simulink model "cddDcmApp"  (R2022b)
settings: Solver=FixedStepDiscrete  StartTime=0.0  StopTime=10.0  FixedStep=0.02  RelTol=1e-3
undefined symbols (not defined in the file or in its folder): CCPFaultType, DcChlType, HFAChlType, IcuChannelType, MtrRoutStaEnum, P_SignalPartType, P_Std_ReturnType, PowerChlType, SrsAdChlType, VGAChlType
(part 4 of 13)

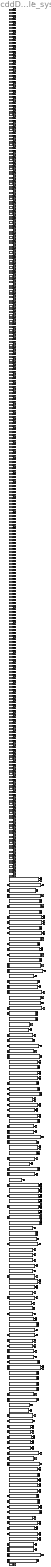
== system cddDcmApp/cddDcmApp_20ms_Runnable_sys ==
  AACAirClngErrStsNgcc_AACAirClngErrStsNgcc_read : Inport
  AACAirClngStsNgcc_AACAirClngStsNgcc_read : Inport  Port=2
  AfuFrameSts_AfuFrameSts_read : Inport  Port=3
  AFUGenErr_AFUGenErr_read : Inport  Port=4
  AirClngErrSts_AirClngErrSts_read : Inport  Port=5
  AirClngSts_AirClngSts_read : Inport  Port=6
  AirFragFanErr_AirFragFanErr_read : Inport  Port=7
  AirFragMot1Err_AirFragMot1Err_read : Inport  Port=8
  AirFragMot2Err_AirFragMot2Err_read : Inport  Port=9
  AirFragMot3Err_AirFragMot3Err_read : Inport  Port=10
  AirFragMot4Err_AirFragMot4Err_read : Inport  Port=11
  AirFragMot5Err_AirFragMot5Err_read : Inport  Port=12
  AirFragOvrVoltErr_AirFragOvrVoltErr_read : Inport  Port=13
  AirFragTempErr_AirFragTempErr_read : Inport  Port=14
  AirFragUndVoltErr_AirFragUndVoltErr_read : Inport  Port=15
  AirTFromHeatgEstimdFirstLeForCCM_AirTFromHeatgEstimdFirstLeForCCM_read : Inport  Port=16
  AirTFromHeatgEstimdFirstRiForCCM_AirTFromHeatgEstimdFirstRiForCCM_read : Inport  Port=17
  AirTFromHeatgEstimdForCCMForRead_AirTFromHeatgEstimdForCCMForRead_read : Inport  Port=18
  AirTFromHeatgEstimdReForCCM_AirTFromHeatgEstimdReForCCM_read : Inport  Port=19
  AirTFromHeatgEstimdSecLeForCCM_AirTFromHeatgEstimdSecLeForCCM_read : Inport  Port=20
  AiuFrameSts_AiuFrameSts_read : Inport  Port=21
  AmbTFildForClimaNgcc_AmbTFildForClima_read : Inport  Port=22
  AmbTRaw_AmbTRaw_read : Inport  Port=23
  AQSAirQuality_AQSAirQuality_read : Inport  Port=24
  AQSCOxstatus_AQSCOxstatus_read : Inport  Port=25
  AqsFrameSts_AqsFrameSts_read : Inport  Port=26
  AQSNH3status_AQSNH3status_read : Inport  Port=27
  AQSNoxStatus_AQSNoxStatus_read : Inport  Port=28
  BkpOfDstTrvld_BkpOfDstTrvld_read : Inport  Port=29
  BkpOfDstTrvld_BkpOfDstTrvld_IsUpdated_read : Inport  Port=30
  CarCfgForClimaCtrl_CarCfgForAirClng_read : Inport  Port=31
  CarCfgForClimaCtrl_CarCfgForAirClnrAdv_read : Inport  Port=32
  CarCfgForClimaCtrl_CarCfgForAirFrag_read : Inport  Port=33
  CarCfgForClimaCtrl_CarCfgForAirMtrlSnsr_read : Inport  Port=34
  CarCfgForClimaCtrl_CarCfgForAirQly_read : Inport  Port=35
  CarCfgForClimaCtrl_CarCfgForAmntOfSeat_read : Inport  Port=36
  CarCfgForClimaCtrl_CarCfgForAutDemst_read : Inport  Port=37
  CarCfgForClimaCtrl_CarCfgForAuxWtrPmp_read : Inport  Port=38
  CarCfgForClimaCtrl_CarCfgForBRAND_read : Inport  Port=39
  CarCfgForClimaCtrl_CarCfgForBypVlvInHvac_read : Inport  Port=40
  CarCfgForClimaCtrl_CarCfgForCabinOxgenSupply_read : Inport  Port=41
  CarCfgForClimaCtrl_CarCfgForClsVlvInHvac_read : Inport  Port=42
  CarCfgForClimaCtrl_CarCfgForCmprAcTyp_read : Inport  Port=43
  CarCfgForClimaCtrl_CarCfgForCmptmtClng_read : Inport  Port=44
  CarCfgForClimaCtrl_CarCfgForElectricLiftingModule_read : Inport  Port=45
  CarCfgForClimaCtrl_CarCfgForElecVentAdjmt_read : Inport  Port=46
  CarCfgForClimaCtrl_CarCfgForExtVentnClimaCtrl_read : Inport  Port=47
  CarCfgForClimaCtrl_CarCfgForFlcTyp_read : Inport  Port=48
  CarCfgForClimaCtrl_CarCfgForFloatingDriving_read : Inport  Port=49
  CarCfgForClimaCtrl_CarCfgForFoldCSD_read : Inport  Port=50
  CarCfgForClimaCtrl_CarCfgForFuTyp_read : Inport  Port=51
  CarCfgForClimaCtrl_CarCfgForGlassTyp_read : Inport  Port=52
  CarCfgForClimaCtrl_CarCfgForHeadRadar_read : Inport  Port=53
  CarCfgForClimaCtrl_CarCfgForHeatPmp_read : Inport  Port=54
  CarCfgForClimaCtrl_CarCfgForHeatrDurgDrvg_read : Inport  Port=55
  CarCfgForClimaCtrl_CarCfgForHeatrTyp_read : Inport  Port=56
  CarCfgForClimaCtrl_CarCfgForHvacFilTyp_read : Inport  Port=57
  CarCfgForClimaCtrl_CarCfgForHvacFlrSnsrForT_read : Inport  Port=58
  CarCfgForClimaCtrl_CarCfgForHvacHeatrSnsrT_read : Inport  Port=59
  CarCfgForClimaCtrl_CarCfgForHvacRe_read : Inport  Port=60
  CarCfgForClimaCtrl_CarCfgForHvacTyp_read : Inport  Port=61
  CarCfgForClimaCtrl_CarCfgForInteriorViewCamera_read : Inport  Port=62
  CarCfgForClimaCtrl_CarCfgForLifeDetection_read : Inport  Port=63
  CarCfgForClimaCtrl_CarCfgForLoVoltElecCfg_read : Inport  Port=64
  CarCfgForClimaCtrl_CarCfgForNavSysandIntnmap_read : Inport  Port=65
  CarCfgForClimaCtrl_CarCfgForNrOfDoor_read : Inport  Port=66
  CarCfgForClimaCtrl_CarCfgForPipeInFuTank_read : Inport  Port=67
  CarCfgForClimaCtrl_CarCfgForPowerSeats_read : Inport  Port=68
  CarCfgForClimaCtrl_CarCfgForPreClng_read : Inport  Port=69
  CarCfgForClimaCtrl_CarCfgForPrkgCDSNotAvl_read : Inport  Port=70
  CarCfgForClimaCtrl_CarCfgForPrkgClima_read : Inport  Port=71
  CarCfgForClimaCtrl_CarCfgForPrpsnTyp_read : Inport  Port=72
  CarCfgForClimaCtrl_CarCfgForPTCInFrontHVAC_read : Inport  Port=73
  CarCfgForClimaCtrl_CarCfgForRemEngStrtPreCdn_read : Inport  Port=74
  CarCfgForClimaCtrl_CarCfgForRoofHatch_read : Inport  Port=75
  CarCfgForClimaCtrl_CarCfgForSeatHeatgCal_read : Inport  Port=76
  CarCfgForClimaCtrl_CarCfgForSeatHeatgForRowFirst_read : Inport  Port=77
  CarCfgForClimaCtrl_CarCfgForSeatHeatgForRowSec_read : Inport  Port=78
  CarCfgForClimaCtrl_CarCfgForSeatVentnForRowFirst_read : Inport  Port=79
  CarCfgForClimaCtrl_CarCfgForSeatVentnForRowSec_read : Inport  Port=80
  CarCfgForClimaCtrl_CarCfgForSecRowVentnCenter_read : Inport  Port=81
  CarCfgForClimaCtrl_CarCfgForSecRowVentnSide_read : Inport  Port=82
  CarCfgForClimaCtrl_CarCfgForSmallFlowCyc_read : Inport  Port=83
  CarCfgForClimaCtrl_CarCfgForSmkSnsr_read : Inport  Port=84
  CarCfgForClimaCtrl_CarCfgForSnsrForCmptmtT_read : Inport  Port=85
  CarCfgForClimaCtrl_CarCfgForSnsrHumPrsnt_read : Inport  Port=86
  CarCfgForClimaCtrl_CarCfgForStartDevice_read : Inport  Port=87
  CarCfgForClimaCtrl_CarCfgForSteerWhlHeatg_read : Inport  Port=88
  CarCfgForClimaCtrl_CarCfgForSteerWhlPosn_read : Inport  Port=89
  CarCfgForClimaCtrl_CarCfgForSunSnsr_read : Inport  Port=90
  CarCfgForClimaCtrl_CarCfgForTelmNodTyp_read : Inport  Port=91
  CarCfgForClimaCtrl_CarCfgForTEMPEREDSTORAGEBOX_read : Inport  Port=92
  CarCfgForClimaCtrl_CarCfgForThermMngt_read : Inport  Port=93
  CarCfgForClimaCtrl_CarCfgForVehTyp_read : Inport  Port=94
  CarCfgForClimaCtrl_CarCfgForVentLiftMotorExiSts_read : Inport  Port=95
  CarCfgForClimaCtrl_CarCfgForVgaMotorExistSts_read : Inport  Port=96
  CarCfgForClimaCtrl_CarCfgForWadingAssistSystem_read : Inport  Port=97
  CarCfgForClimaCtrl_CarCfgForWindHeatd_read : Inport  Port=98
  CarCfgForClimaCtrl_CarCfgWindFollowHumanMov_read : Inport  Port=99
  CarTiGlb_CarTiGlb_read : Inport  Port=100
  CarTiGlb_CarTiGlb_IsUpdated_read : Inport  Port=101
  CcmIntCo2Lvl_CcmIntCo2Lvl_read : Inport  Port=102
  CCMLIN1frameSts_CCMLIN1frameSts_read : Inport  Port=103
  CCMLIN2frameSts_CCMLIN2frameSts_read : Inport  Port=104
  CCMLIN3frameSts_CCMLIN3frameSts_read : Inport  Port=105
  CdsFrameSts_CdsFrameSts_read : Inport  Port=106
  ClimaObsActv_ClimaObsActv_read : Inport  Port=107
  CmpmtInAirPmAQI_CmpmtInAirPmAQI_read : Inport  Port=108
  CmpmtInAirPmDnsty_CmpmtInAirPmDnsty_read : Inport  Port=109
  CmptmtAirTEstimdAtRowFirst_CmptmtAirTEstimdAtRowFirst_read : Inport  Port=110
  CmptmtAirTEstimdAtRowFirstIni_CmptmtAirTEstimdAtRowFirstIni_read : Inport  Port=111
  CmptmtAirTEstimdAtRowSec_CmptmtAirTEstimdAtRowSec_read : Inport  Port=112
  CmptmtAirTEstimdAtRowSecIni_CmptmtAirTEstimdAtRowSecIni_read : Inport  Port=113
  CmptmtAirTEstimdFirstNvRamIni_CmptmtAirTEstimdFirstNvRamIni_read : Inport  Port=114
  CmptmtAirTEstimdSecNvRamIni_CmptmtAirTEstimdSecNvRamIni_read : Inport  Port=115
  CmptmtFrntWindDewT_CmptmtFrntWindDewT_read : Inport  Port=116
  CmptmtFrntWindT_CmptmtFrntWindT_read : Inport  Port=117
  CmptmtOxygenConcVluNgcc_CmptmtOxygenConcVluNgcc_read : Inport  Port=118
  CmptmtRelHum_CmptmtRelHum_read : Inport  Port=119
  CmptmtRelHum_CmptmtRelHum_IsUpdated_read : Inport  Port=120
  CmptmtTRearRaw_CmptmtTRearRaw_read : Inport  Port=121
  CoupldCtrlErrAtRowFirstLe_CoupldCtrlErrAtRowFirstLe_read : Inport  Port=122
  CoupldCtrlErrAtRowFirstRi_CoupldCtrlErrAtRowFirstRi_read : Inport  Port=123
  CoupldCtrlErrAtRowSecLe_CoupldCtrlErrAtRowSecLe_read : Inport  Port=124
  CoupldCtrlErrAtRowSecRi_CoupldCtrlErrAtRowSecRi_read : Inport  Port=125
  DcMotorCurPosVol_DcMotorCurPosVol_read : Inport  Port=126
  EngT_Ngcc_EngT_read : Inport  Port=127
  EvaprTFrntFildForClima_EvaprTFrntFildForClima_read : Inport  Port=128
  EvaprTReFildForClima_EvaprTReFildForClima_read : Inport  Port=129
  HmiHvacFanLvlFrnt_HmiHvacFanLvlFrnt_read : Inport  Port=130
  HmiHvacFanLvlRe_HmiHvacFanLvlRe_read : Inport  Port=131
  HvacAirTEstimdAtRowFirstLe_HvacAirTEstimdAtRowFirstLe_read : Inport  Port=132
  HvacAirTEstimdAtRowFirstRi_HvacAirTEstimdAtRowFirstRi_read : Inport  Port=133
  HvacAirTEstimdAtRowSecLe_HvacAirTEstimdAtRowSecLe_read : Inport  Port=134
  HvacAirTEstimdAtRowSecRi_HvacAirTEstimdAtRowSecRi_read : Inport  Port=135
  HvacAirTReqAtRowFirstLe_HvacAirTReqAtRowFirstLe_read : Inport  Port=136
  HvacAirTReqAtRowFirstRi_HvacAirTReqAtRowFirstRi_read : Inport  Port=137
  HvacAirTReqAtRowSecLe_HvacAirTReqAtRowSecLe_read : Inport  Port=138
  HvacAirTReqAtRowSecRi_HvacAirTReqAtRowSecRi_read : Inport  Port=139
  HvacCfgForClimaCtrl_HvacCfgForAQSTypes_read : Inport  Port=140
  HvacCfgForClimaCtrl_HvacCfgForHvacFrontBlowerType_read : Inport  Port=141
  HvacCfgForClimaCtrl_HvacCfgForHvacLowSideRETXVESts_read : Inport  Port=142
  HvacCfgForClimaCtrl_HvacCfgForHvacMiscExistSts_read : Inport  Port=143
  HvacCfgForClimaCtrl_HvacCfgForHvacMotorExistSts_read : Inport  Port=144
  HvacCfgForClimaCtrl_HvacCfgForHvacReBlowerType_read : Inport  Port=145
  HvacCfgForClimaCtrl_HvacCfgForHvacReVentPstn_read : Inport  Port=146
  HvacCfgForClimaCtrl_HvacCfgForHvacSensorExistSts_read : Inport  Port=147
  HvacCfgForClimaCtrl_HvacCfgForHvacSnsrRCmptmtESts_read : Inport  Port=148
  HvacCfgForClimaCtrl_HvacCfgForHvacSnsrReHexESts_read : Inport  Port=149
  HvacFanSet3HvacFanSpd1_HvacFanSet3HvacFanSpd1_read : Inport  Port=150
  HvacFanSts2HvacFanBattI2_HvacFanSts2HvacFanBattI2_read : Inport  Port=151
  HvacFanSts2HvacFanBattU2_HvacFanSts2HvacFanBattU2_read : Inport  Port=152
  HvacFanSts2HvacFanMotU2_HvacFanSts2HvacFanMotU2_read : Inport  Port=153
  HvacFanSts2HvacFanReSts1_HvacFanSts2HvacFanReSts1_read : Inport  Port=154
  HvacFanSts2HvacFanReSts2_HvacFanSts2HvacFanReSts2_read : Inport  Port=155
  HvacFanSts3HvacFanBattI_HvacFanSts3HvacFanBattI_read : Inport  Port=156
  HvacFanSts3HvacFanBattU_HvacFanSts3HvacFanBattU_read : Inport  Port=157
  HvacFanSts3HvacFanCircShoOrMotBlkd1_HvacFanSts3HvacFanCircShoOrMotBlkd1_read : Inport  Port=158
  HvacFanSts3HvacFanDiagFlag1_HvacFanSts3HvacFanDiagFlag1_read : Inport  Port=159
  HvacFanSts3HvacFanILimn_HvacFanSts3HvacFanILimn_read : Inport  Port=160
  HvacFanSts3HvacFanIntErr1_HvacFanSts3HvacFanIntErr1_read : Inport  Port=161
  HvacFanSts3HvacFanSpdFd_HvacFanSts3HvacFanSpdFd_read : Inport  Port=162
  HvacFanSts3HvacFanTErr_HvacFanSts3HvacFanTErr_read : Inport  Port=163
  HvacFanSts3HvDetd_HvacFanSts3HvDetd_read : Inport  Port=164
  HvacFanSts3OverCurrErr_HvacFanSts3OverCurrErr_read : Inport  Port=165
  HvacFanSts3UDetdLo_HvacFanSts3UDetdLo_read : Inport  Port=166
  HvacFanVoltReqFrnt_HvacFanVoltReqFrnt_read : Inport  Port=167
  HvacFanVoltReqRe_HvacFanVoltReqRe_read : Inport  Port=168
  HvacLpmUFbRe_HvacLpmUFbRe_read : Inport  Port=169
  HvacPwm2MosVFbFrnt_HvacPwm2MosVFbFrnt_read : Inport  Port=170
  HvacPwmSignErr_HvacPwmSignErr_read : Inport  Port=171
  InPm25FanErr_InPm25FanErr_read : Inport  Port=172
  InPm25SensrIntErr_InPm25SensrIntErr_read : Inport  Port=173
  InPm25SnrTempErr_InPm25SnrTempErr_read : Inport  Port=174
  InPm25SnrVoltgRangErr_InPm25SnrVoltgRangErr_read : Inport  Port=175
  InPm25SnsrElecErr_InPm25SnsrElecErr_read : Inport  Port=176
  IntCo2Conc_IntCo2Conc_read : Inport  Port=177
  IntCo2Lvl_IntCo2Lvl_read : Inport  Port=178
  LdAdVal_CurrAdVal_read : Inport  Port=179
  LdAdVal_ValidAdVal_read : Inport  Port=180
  MemReadForCenVentLiftModSts_MemReadForCenVentLiftModSts_read : Inport  Port=181
  MemReadForClimaEgyMgrForDID_MemReadForClimaEgyMgrForDID_read : Inport  Port=182
  NvMBlockCCMLoadDataArray_NvMBlockCCMLoadDataArray_read : Inport  Port=183
  NvMBlockDcMtrCalDataArray_NvMBlockDcMtrCalDataArray_read : Inport  Port=184
  ObsvrAirTOffsFromWindTIni_ObsvrAirTOffsFromWindTIni_read : Inport  Port=185
  ObsvrCmptmtTIninActv_ObsvrCmptmtTIninActv_read : Inport  Port=186
  ObsvrCmptmtTRamRxInt_ObsvrCmptmtTRamRxInt_read : Inport  Port=187
  ObsvrInCarTFrntIni_ObsvrInCarTFrntIni_read : Inport  Port=188
  ObsvrInCarTRearIni_ObsvrInCarTRearIni_read : Inport  Port=189
  ObsvrIntrMtrlTIni_ObsvrIntrMtrlTIni_read : Inport  Port=190
  OGMIntErrNgcc_OGMIntErrNgcc_read : Inport  Port=191
  OGMOvrVoltRangErrNgcc_OGMOvrVoltRangErrNgcc_read : Inport  Port=192
  OGMTempErrNgcc_OGMTempErrNgcc_read : Inport  Port=193
  OGMUndVoltRangErrNgcc_OGMUndVoltRangErrNgcc_read : Inport  Port=194
  OutdAirQly_OutdAirQly_read : Inport  Port=195
  OxygenConcSnsrElecErrNgcc_OxygenConcSnsrElecErrNgcc_read : Inport  Port=196
  OxygenConcSnsrIntErrNgcc_OxygenConcSnsrIntErrNgcc_read : Inport  Port=197
  OxygenConcSnsrOvrVoltRangErrNgcc_OxygenConcSnsrOvrVoltRangErrNgcc_read : Inport  Port=198
  OxygenConcSnsrTempErrNgcc_OxygenConcSnsrTempErrNgcc_read : Inport  Port=199
  OxygenConcSnsrUndVoltRangErrNgcc_OxygenConcSnsrUndVoltRangErrNgcc_read : Inport  Port=200
  PmsiFrameSts_PmsiFrameSts_read : Inport  Port=201
  ReEvapVlvSts_ReEvapVlvSts_read : Inport  Port=202
  ReHvacActrElecErr_ReHvacActrElecErr_read : Inport  Port=203
  ReHvacActrOvrTempErr_ReHvacActrOvrTempErr_read : Inport  Port=204
  ReHvacActrVoltErr_ReHvacActrVoltErr_read : Inport  Port=205
  RelHumSnsrRelHum_RelHumSnsrRelHum_read : Inport  Port=206
  RhfaFrameSts_RhfaFrameSts_read : Inport  Port=207
  SensorAdValue_SensorAdValue_read : Inport  Port=208
  SrsAdVal_CurrAdVal_read : Inport  Port=209
  SrsAdVal_ValidAdVal_read : Inport  Port=210
  SunData_SunData_read : Inport  Port=211
  SWVersion_SWVersion_read : Inport  Port=212
  SysServCarCfgSts_SysServCarID_read : Inport  Port=213
  SysServCarCfgSts_SysServCCPAQSSts_read : Inport  Port=214
  SysServCarCfgSts_SysServCCPBGMNum_read : Inport  Port=215
  SysServCarCfgSts_SysServCCPHEXSensorSts_read : Inport  Port=216
  SysServCarCfgSts_SysServCCPLin2AFUSts_read : Inport  Port=217
  SysServCarCfgSts_SysServCCPLin2AIUSts_read : Inport  Port=218
  SysServCarCfgSts_SysServCCPLin2AQSSts_read : Inport  Port=219
  SysServCarCfgSts_SysServCCPLin2CDSSts_read : Inport  Port=220
  SysServCarCfgSts_SysServCCPLin2PMSISts_read : Inport  Port=221
  SysServCarCfgSts_SysServCCPLin3VGASts_read : Inport  Port=222
  SysServCarCfgSts_SysServDUAirVentSnrSts_read : Inport  Port=223
  SysServCarCfgSts_SysServDUBlowerPwmSignSts_read : Inport  Port=224
  SysServCarCfgSts_SysServDUBlowerPwmSignTypeSts_read : Inport  Port=225
  SysServCarCfgSts_SysServDUBlowerPwmSts_read : Inport  Port=226
  SysServCarCfgSts_SysServDUDCDefrostSts_read : Inport  Port=227
  SysServCarCfgSts_SysServDUDCFLMdeSts_read : Inport  Port=228
  SysServCarCfgSts_SysServDUDCModeSts_read : Inport  Port=229
  SysServCarCfgSts_SysServDUDCRecSts_read : Inport  Port=230
  SysServCarCfgSts_SysServDUDCSModeSts_read : Inport  Port=231
  SysServCarCfgSts_SysServDUDCSTLSts_read : Inport  Port=232
  SysServCarCfgSts_SysServDUDCTLSts_read : Inport  Port=233
  SysServCarCfgSts_SysServDUDCTRSts_read : Inport  Port=234
  SysServCarCfgSts_SysServDUFLFootSts_read : Inport  Port=235
  SysServCarCfgSts_SysServDUFLVentSts_read : Inport  Port=236
  SysServCarCfgSts_SysServDUFRFootSts_read : Inport  Port=237
  SysServCarCfgSts_SysServDUFRVentSts_read : Inport  Port=238
  SysServCarCfgSts_SysServDUIncarMotorSts_read : Inport  Port=239
  SysServCarCfgSts_SysServDULin1HBMFSts_read : Inport  Port=240
  SysServCarCfgSts_SysServDULin4HFAFSts_read : Inport  Port=241
  SysServCarCfgSts_SysServDULin4HFAFStsH_read : Inport  Port=242
  SysServCarCfgSts_SysServDUReBlowerPwmSignSts_read : Inport  Port=243
  SysServCarCfgSts_SysServDUReBlowerPwmSts_read : Inport  Port=244
  SysServCarCfgSts_SysServDUSFLFootSts_read : Inport  Port=245
  SysServCarCfgSts_SysServDUSFLVentSts_read : Inport  Port=246
  SysServCarCfgSts_SysServDUSFRFootSts_read : Inport  Port=247
  SysServCarCfgSts_SysServDUSFRVentSts_read : Inport  Port=248
  SysServCarCfgSts_SysServDUSnsrForFCmptmtTSts_read : Inport  Port=249
  SysServCarCfgSts_SysServDUSnsrForRair_read : Inport  Port=250
  SysServCarCfgSts_SysServDUSnsrForRCmptmtTSts_read : Inport  Port=251
  SysServCarCfgSts_SysServFrntHEXSnsrPosn_read : Inport  Port=252
  SysServCarCfgSts_SysServLowSideRETXVSts_read : Inport  Port=253
  SysServCarCfgSts_SysServReHEXSnsrPosn_read : Inport  Port=254
  SysServCarCfgSts_SysServRHFASts_read : Inport  Port=255
  SysServCarCfgSts_SysServSnsrForFCmptmtTType_read : Inport  Port=256
  TestRunCriteriaTRC_TestRunCriteriaTRC_read : Inport  Port=257
  TiOfDeactvnForClimaObs_TiOfDeactvnForClimaObs_read : Inport  Port=258
  VbmrFrameSts_VbmrFrameSts_read : Inport  Port=259
  VehBattU_VehBattU_read : Inport  Port=260
  VehBattU_VehBattU_IsUpdated_read : Inport  Port=261
  VehModMngtGlbSafe1_VehModMngtGlbSafe1_read : Inport  Port=262
  VehModMngtGlbSafe1_VehModMngtGlbSafe1_IsUpdated_read : Inport  Port=263
  VentnActr01ElecErr_VentnActr01ElecErr_read : Inport  Port=264
  VentnActr01OvrTempErr_VentnActr01OvrTempErr_read : Inport  Port=265
  VentnActr01VoltErr_VentnActr01VoltErr_read : Inport  Port=266
  VentnActr02ElecErr_VentnActr02ElecErr_read : Inport  Port=267
  VentnActr02OvrTempErr_VentnActr02OvrTempErr_read : Inport  Port=268
  VentnActr02VoltErr_VentnActr02VoltErr_read : Inport  Port=269
  VentnActr03ElecErr_VentnActr03ElecErr_read : Inport  Port=270
  VentnActr03OvrTempErr_VentnActr03OvrTempErr_read : Inport  Port=271
  VentnActr03VoltErr_VentnActr03VoltErr_read : Inport  Port=272
  VentnActr04ElecErr_VentnActr04ElecErr_read : Inport  Port=273
  VentnActr04OvrTempErr_VentnActr04OvrTempErr_read : Inport  Port=274
  VentnActr04VoltErr_VentnActr04VoltErr_read : Inport  Port=275
  VentnActr05ElecErr_VentnActr05ElecErr_read : Inport  Port=276
  VentnActr05OvrTempErr_VentnActr05OvrTempErr_read : Inport  Port=277
  VentnActr05VoltErr_VentnActr05VoltErr_read : Inport  Port=278
  VentnActr06ElecErr_VentnActr06ElecErr_read : Inport  Port=279
  VentnActr06OvrTempErr_VentnActr06OvrTempErr_read : Inport  Port=280
  VentnActr06VoltErr_VentnActr06VoltErr_read : Inport  Port=281
  VentnActr07ElecErr_VentnActr07ElecErr_read : Inport  Port=282
  VentnActr07OvrTempErr_VentnActr07OvrTempErr_read : Inport  Port=283
  VentnActr07VoltErr_VentnActr07VoltErr_read : Inport  Port=284
  VentnActr08ElecErr_VentnActr08ElecErr_read : Inport  Port=285
  VentnActr08OvrTempErr_VentnActr08OvrTempErr_read : Inport  Port=286
  VentnActr08VoltErr_VentnActr08VoltErr_read : Inport  Port=287
  VentnActr09ElecErr_VentnActr09ElecErr_read : Inport  Port=288
  VentnActr09OvrTempErr_VentnActr09OvrTempErr_read : Inport  Port=289
  VentnActr09VoltErr_VentnActr09VoltErr_read : Inport  Port=290
  BswLifeCycle_LifeCycle_read : Inport  Port=291
  function : TriggerPort  TriggerType=function-call  VariantControl=(inherit)  SampleTimeType=periodic  SampleTime=-1
  BatParaService_GetBatteryVolValue : FunctionCaller  FunctionPrototype=[BatVolValue,ERR] = BatParaService_GetBatteryVolValue()  OutputArgumentSpecifications=P_BatteryVolValue, P_Std_ReturnType
  DcMotorIOCtrlService_FreezeMotorCurrentPosition : FunctionCaller  FunctionPrototype=ERR = DcMotorIOCtrlService_FreezeMotorCurrentPosition(Channel)  InputArgumentSpecifications=DcChlType(0)  OutputArgumentSpecifications=P_Std_ReturnType
  DcMotorIOCtrlService_MotorRetCtlToECU : FunctionCaller  FunctionPrototype=ERR = DcMotorIOCtrlService_MotorRetCtlToECU(Channel)  InputArgumentSpecifications=DcChlType(0)  OutputArgumentSpecifications=P_Std_ReturnType
  DcMotorIOCtrlService_MotorShortTermAdj : FunctionCaller  FunctionPrototype=ERR = DcMotorIOCtrlService_MotorShortTermAdj(Channel,Postion)  InputArgumentSpecifications=DcChlType(0), uint16(1)  OutputArgumentSpecifications=P_Std_ReturnType
  DcMotorParaService_DcMotorAllowSleepSts : FunctionCaller  FunctionPrototype=[Status,ERR] = DcMotorParaService_DcMotorAllowSleepSts()  OutputArgumentSpecifications=uint8(1), P_Std_ReturnType
  DcMotorParaService_GetMotorActualPositon : FunctionCaller  FunctionPrototype=[Postion,ERR] = DcMotorParaService_GetMotorActualPositon(Channel)  InputArgumentSpecifications=DcChlType(0)  OutputArgumentSpecifications=uint16(1), P_Std_ReturnType
  DcMotorParaService_GetMotorCailLearnSts : FunctionCaller  FunctionPrototype=[Status,ERR] = DcMotorParaService_GetMotorCailLearnSts()  OutputArgumentSpecifications=uint8(1), P_Std_ReturnType
  DcMotorParaService_GetMotorCalibrationStatus : FunctionCaller  FunctionPrototype=[Status,ERR] = DcMotorParaService_GetMotorCalibrationStatus(Channel)  InputArgumentSpecifications=DcChlType(0)  OutputArgumentSpecifications=uint8(1), P_Std_ReturnType
  DcMotorParaService_GetMotorDidWorkingStatus : FunctionCaller  FunctionPrototype=[Status,ERR] = DcMotorParaService_GetMotorDidWorkingStatus(Channel)  InputArgumentSpecifications=DcChlType(0)  OutputArgumentSpecifications=uint8(1), P_Std_ReturnType
  DcMotorParaService_GetMotorEndPosition : FunctionCaller  FunctionPrototype=[Postion,ERR] = DcMotorParaService_GetMotorEndPosition(Channel)  InputArgumentSpecifications=DcChlType(0)  OutputArgumentSpecifications=uint16(1), P_Std_ReturnType
  DcMotorParaService_GetMotorRequestPercent : FunctionCaller  FunctionPrototype=[Position,ERR] = DcMotorParaService_GetMotorRequestPercent(Channel)  InputArgumentSpecifications=DcChlType(0)  OutputArgumentSpecifications=uint16(1), P_Std_ReturnType
  DcMotorParaService_GetMotorRoutineCailSts : FunctionCaller  FunctionPrototype=[Status,ERR] = DcMotorParaService_GetMotorRoutineCailSts()  OutputArgumentSpecifications=uint8(1), P_Std_ReturnType
  DcMotorParaService_GetMotorRunTime : FunctionCaller  FunctionPrototype=[Runtime,ERR] = DcMotorParaService_GetMotorRunTime(Channel)  InputArgumentSpecifications=DcChlType(0)  OutputArgumentSpecifications=uint32(1), P_Std_ReturnType
  DcMotorParaService_GetMotorWorkingStatus : FunctionCaller  FunctionPrototype=[Status,ERR] = DcMotorParaService_GetMotorWorkingStatus(Channel)  InputArgumentSpecifications=DcChlType(0)  OutputArgumentSpecifications=uint8(1), P_Std_ReturnType
  DcMotorParaService_GetMotorZeroPosition : FunctionCaller  FunctionPrototype=[Position,ERR] = DcMotorParaService_GetMotorZeroPosition(Channel)  InputArgumentSpecifications=DcChlType(0)  OutputArgumentSpecifications=uint16(1), P_Std_ReturnType
  DcMotorParaService_GetRamAirErrorStatus : FunctionCaller  FunctionPrototype=[Status,ERR] = DcMotorParaService_GetRamAirErrorStatus()  OutputArgumentSpecifications=uint8(1), P_Std_ReturnType
  DcMotorParaService_SetMotorEndPosition : FunctionCaller  FunctionPrototype=ERR = DcMotorParaService_SetMotorEndPosition(Channel,Postion)  InputArgumentSpecifications=DcChlType(0), uint16(1)  OutputArgumentSpecifications=P_Std_ReturnType
  DcMotorParaService_SetMotorRequestPercent : FunctionCaller  FunctionPrototype=ERR = DcMotorParaService_SetMotorRequestPercent(Channel,Position)  InputArgumentSpecifications=DcChlType(0), uint16(1)  OutputArgumentSpecifications=P_Std_ReturnType
  DcMotorParaService_SetMotorRoutineCail : FunctionCaller  FunctionPrototype=ERR = DcMotorParaService_SetMotorRoutineCail()  OutputArgumentSpecifications=P_Std_ReturnType
  DcMotorParaService_SetMotorRoutineCailSts : FunctionCaller  FunctionPrototype=ERR = DcMotorParaService_SetMotorRoutineCailSts(Status)  InputArgumentSpecifications=uint8(1)  OutputArgumentSpecifications=P_Std_ReturnType
  DcMotorParaService_SetMotorRunTime : FunctionCaller  FunctionPrototype=ERR = DcMotorParaService_SetMotorRunTime(Channel,Runtime)  InputArgumentSpecifications=DcChlType(0), uint32(1)  OutputArgumentSpecifications=P_Std_ReturnType
  DcMotorParaService_SetMotorZeroPosition : FunctionCaller  FunctionPrototype=ERR = DcMotorParaService_SetMotorZeroPosition(Channel,Position)  InputArgumentSpecifications=DcChlType(0), uint16(1)  OutputArgumentSpecifications=P_Std_ReturnType
  Ground_{1..94} (94 alike) : Ground
  HbmfParaService_FrntBlwrspdLIN1_FreezeCurrentState : FunctionCaller  FunctionPrototype=ERR = HbmfParaService_FrntBlwrspdLIN1_FreezeCurrentState()  OutputArgumentSpecifications=P_Std_ReturnType
  HbmfParaService_FrntBlwrspdLIN1_ReturnControlToECU : FunctionCaller  FunctionPrototype=ERR = HbmfParaService_FrntBlwrspdLIN1_ReturnControlToECU()  OutputArgumentSpecifications=P_Std_ReturnType
  HbmfParaService_FrntBlwrspdLIN1_ShortTermAdjustment : FunctionCaller  FunctionPrototype=ERR = HbmfParaService_FrntBlwrspdLIN1_ShortTermAdjustment(Data)  InputArgumentSpecifications=uint8([1])  OutputArgumentSpecifications=P_Std_ReturnType
  HbmfParaService_GetHbmfDvAsmPartNr : FunctionCaller  FunctionPrototype=[Data,ERR] = HbmfParaService_GetHbmfDvAsmPartNr(Part)  InputArgumentSpecifications=P_SignalPartType  OutputArgumentSpecifications=uint8([1]), P_Std_ReturnType
  HbmfParaService_GetHbmfPrvEcuSerNr : FunctionCaller  FunctionPrototype=[Data,ERR] = HbmfParaService_GetHbmfPrvEcuSerNr()  OutputArgumentSpecifications=uint8([1]), P_Std_ReturnType
  HfaCaliService_SetAllCalibrate : FunctionCaller  FunctionPrototype=ERR = HfaCaliService_SetAllCalibrate()  OutputArgumentSpecifications=P_Std_ReturnType
  HfaCaliService_SetAllParaInit : FunctionCaller  FunctionPrototype=ERR = HfaCaliService_SetAllParaInit()  OutputArgumentSpecifications=P_Std_ReturnType
  HfaCaliService_SetMotorCalibrate : FunctionCaller  FunctionPrototype=ERR = HfaCaliService_SetMotorCalibrate(Channel)  InputArgumentSpecifications=HFAChlType(0)  OutputArgumentSpecifications=P_Std_ReturnType
  HfaCaliService_SetMotorParaInit : FunctionCaller  FunctionPrototype=ERR = HfaCaliService_SetMotorParaInit(Channel)  InputArgumentSpecifications=HFAChlType(0)  OutputArgumentSpecifications=P_Std_ReturnType
  HfaIOCtrlService_FreezeMotorCurrentPosition : FunctionCaller  FunctionPrototype=ERR = HfaIOCtrlService_FreezeMotorCurrentPosition(Channel)  InputArgumentSpecifications=HFAChlType(0)  OutputArgumentSpecifications=P_Std_ReturnType
  HfaIOCtrlService_MotorReturnToECU : FunctionCaller  FunctionPrototype=ERR = HfaIOCtrlService_MotorReturnToECU(Channel)  InputArgumentSpecifications=HFAChlType(0)  OutputArgumentSpecifications=P_Std_ReturnType
  HfaIOCtrlService_MotorShortTermAdj : FunctionCaller  FunctionPrototype=ERR = HfaIOCtrlService_MotorShortTermAdj(Channel,TempValue)  InputArgumentSpecifications=HFAChlType(0), uint16(1)  OutputArgumentSpecifications=P_Std_ReturnType
  HfaParaService_GetAllMotorCaliStatus : FunctionCaller  FunctionPrototype=[Status,ERR] = HfaParaService_GetAllMotorCaliStatus()  OutputArgumentSpecifications=uint8(1), P_Std_ReturnType
  HfaParaService_GetCailRoutineStatus : FunctionCaller  FunctionPrototype=[Status,ERR] = HfaParaService_GetCailRoutineStatus()  OutputArgumentSpecifications=MtrRoutStaEnum(0), P_Std_ReturnType
  HfaParaService_GetFreshRecErrorStatus : FunctionCaller  FunctionPrototype=[Status,ERR] = HfaParaService_GetFreshRecErrorStatus()  OutputArgumentSpecifications=uint8(1), P_Std_ReturnType
  HfaParaService_GetMotorAllowSleepSts : FunctionCaller  FunctionPrototype=[Status,ERR] = HfaParaService_GetMotorAllowSleepSts()  OutputArgumentSpecifications=uint8(1), P_Std_ReturnType
  HfaParaService_GetMotorCaliStatus : FunctionCaller  FunctionPrototype=[Status,ERR] = HfaParaService_GetMotorCaliStatus(Channel)  InputArgumentSpecifications=HFAChlType(0)  OutputArgumentSpecifications=uint8(1), P_Std_ReturnType
  HfaParaService_GetMotorCurrentPosition : FunctionCaller  FunctionPrototype=[Pos,ERR] = HfaParaService_GetMotorCurrentPosition(Channel)  InputArgumentSpecifications=HFAChlType(0)  OutputArgumentSpecifications=uint16(1), P_Std_ReturnType
  HfaParaService_GetMotorDidWorkingStatus : FunctionCaller  FunctionPrototype=[Status,ERR] = HfaParaService_GetMotorDidWorkingStatus(Channel)  InputArgumentSpecifications=HFAChlType(0)  OutputArgumentSpecifications=uint8(1), P_Std_ReturnType
  HfaParaService_GetMotorMoveCnt : FunctionCaller  FunctionPrototype=[Cnt,ERR] = HfaParaService_GetMotorMoveCnt(Channel)  InputArgumentSpecifications=HFAChlType(0)  OutputArgumentSpecifications=uint32(1), P_Std_ReturnType
  HfaParaService_GetMotorReqPosition : FunctionCaller  FunctionPrototype=[Position,ERR] = HfaParaService_GetMotorReqPosition(Channel)  InputArgumentSpecifications=HFAChlType(0)  OutputArgumentSpecifications=uint16(1), P_Std_ReturnType
  HfaParaService_GetMotorStepRange : FunctionCaller  FunctionPrototype=[Range,ERR] = HfaParaService_GetMotorStepRange(Channel)  InputArgumentSpecifications=HFAChlType(0)  OutputArgumentSpecifications=uint16(1), P_Std_ReturnType
  HfaParaService_GetMotorWorkingStatus : FunctionCaller  FunctionPrototype=[Status,ERR] = HfaParaService_GetMotorWorkingStatus(Channel)  InputArgumentSpecifications=HFAChlType(0)  OutputArgumentSpecifications=uint8(1), P_Std_ReturnType
  HfaParaService_GetRamAirErrorStatus : FunctionCaller  FunctionPrototype=[Status,ERR] = HfaParaService_GetRamAirErrorStatus()  OutputArgumentSpecifications=uint8(1), P_Std_ReturnType
  HfaParaService_SetCailRoutineStatus : FunctionCaller  FunctionPrototype=ERR = HfaParaService_SetCailRoutineStatus(Status)  InputArgumentSpecifications=MtrRoutStaEnum(0)  OutputArgumentSpecifications=P_Std_ReturnType
  HfaParaService_SetMotorMoveCnt : FunctionCaller  FunctionPrototype=ERR = HfaParaService_SetMotorMoveCnt(Channel,Cnt)  InputArgumentSpecifications=HFAChlType(0), uint32(1)  OutputArgumentSpecifications=P_Std_ReturnType
  HfaParaService_SetMotorRequestPosition : FunctionCaller  FunctionPrototype=ERR = HfaParaService_SetMotorRequestPosition(Channel,Present)  InputArgumentSpecifications=HFAChlType(0), uint16(1)  OutputArgumentSpecifications=P_Std_ReturnType
  HfaParaService_SetMotorStepRange : FunctionCaller  FunctionPrototype=ERR = HfaParaService_SetMotorStepRange(Channel,Range)  InputArgumentSpecifications=HFAChlType(0), uint16(1)  OutputArgumentSpecifications=P_Std_ReturnType
  HfaRIDService_GetAutoAddreRoutineSts : FunctionCaller  FunctionPrototype=[Status,ERR] = HfaRIDService_GetAutoAddreRoutineSts()  OutputArgumentSpecifications=MtrRoutStaEnum(0), P_Std_ReturnType
  HfaRIDService_GetMotorAutoAddreSts : FunctionCaller  FunctionPrototype=[Status,ERR] = HfaRIDService_GetMotorAutoAddreSts()  OutputArgumentSpecifications=uint8(1), P_Std_ReturnType
  HfaRIDService_SetAutoAddreRoutineSts : FunctionCaller  FunctionPrototype=ERR = HfaRIDService_SetAutoAddreRoutineSts(Status)  InputArgumentSpecifications=MtrRoutStaEnum(0)  OutputArgumentSpecifications=P_Std_ReturnType
  HfaRIDService_SetMotorAutoAddre : FunctionCaller  FunctionPrototype=ERR = HfaRIDService_SetMotorAutoAddre()  OutputArgumentSpecifications=P_Std_ReturnType
  IcuServ_GetDutyCycleValues : FunctionCaller  FunctionPrototype=[DutyCycleValues,ERR] = IcuServ_GetDutyCycleValues(Channel)  InputArgumentSpecifications=IcuChannelType(0)  OutputArgumentSpecifications=P_IcuDutyCycleType, P_Std_ReturnType
  IcuServ_IcuStart : FunctionCaller  FunctionPrototype=ERR = IcuServ_IcuStart()  OutputArgumentSpecifications=P_Std_ReturnType
  IcuServ_SetMaxPwmPeriod : FunctionCaller  FunctionPrototype=ERR = IcuServ_SetMaxPwmPeriod(Channel,MaxPwmPeriod)  InputArgumentSpecifications=IcuChannelType(0), uint16(1)  OutputArgumentSpecifications=P_Std_ReturnType
  Lin2MgrParaServ_AFU_DvAsmPartNr : FunctionCaller  FunctionPrototype=[DataArray,Sta,ERR] = Lin2MgrParaServ_AFU_DvAsmPartNr(Part)  InputArgumentSpecifications=uint8(1)  OutputArgumentSpecifications=uint8([1;1;1;1;1]), uint8(1), P_Std_ReturnType
  Lin2MgrParaServ_AFU_PrvEcuSerNr : FunctionCaller  FunctionPrototype=[DataArray,Sta,ERR] = Lin2MgrParaServ_AFU_PrvEcuSerNr()  OutputArgumentSpecifications=uint8([1;1;1;1;1]), uint8(1), P_Std_ReturnType
  Lin2MgrParaServ_AIU_DvAsmPartNr : FunctionCaller  FunctionPrototype=[DataArray,Sta,ERR] = Lin2MgrParaServ_AIU_DvAsmPartNr(Part)  InputArgumentSpecifications=uint8(1)  OutputArgumentSpecifications=uint8([1;1;1;1;1]), uint8(1), P_Std_ReturnType
  Lin2MgrParaServ_AIU_PrvEcuSerNr : FunctionCaller  FunctionPrototype=[DataArray,Sta,ERR] = Lin2MgrParaServ_AIU_PrvEcuSerNr()  OutputArgumentSpecifications=uint8([1;1;1;1;1]), uint8(1), P_Std_ReturnType
  Lin2MgrParaServ_CDS_DvAsmPartNr : FunctionCaller  FunctionPrototype=[DataArray,Sta,ERR] = Lin2MgrParaServ_CDS_DvAsmPartNr(Part)  InputArgumentSpecifications=uint8(1)  OutputArgumentSpecifications=uint8([1;1;1;1;1]), uint8(1), P_Std_ReturnType
  Lin2MgrParaServ_CDS_PrvEcuSerNr : FunctionCaller  FunctionPrototype=[DataArray,Sta,ERR] = Lin2MgrParaServ_CDS_PrvEcuSerNr()  OutputArgumentSpecifications=uint8([1;1;1;1;1]), uint8(1), P_Std_ReturnType
  Lin2MgrParaServ_PMSI_DvAsmPartNr : FunctionCaller  FunctionPrototype=[DataArray,Sta,ERR] = Lin2MgrParaServ_PMSI_DvAsmPartNr(Part)  InputArgumentSpecifications=uint8(1)  OutputArgumentSpecifications=uint8([1;1;1;1;1]), uint8(1), P_Std_ReturnType
  Lin2MgrParaServ_PMSI_PrvEcuSerNr : FunctionCaller  FunctionPrototype=[DataArray,Sta,ERR] = Lin2MgrParaServ_PMSI_PrvEcuSerNr()  OutputArgumentSpecifications=uint8([1;1;1;1;1]), uint8(1), P_Std_ReturnType
  Lin2ParaService_FreezeVbmrReqPercent : FunctionCaller  FunctionPrototype=ERR = Lin2ParaService_FreezeVbmrReqPercent()  OutputArgumentSpecifications=P_Std_ReturnType
  Lin2ParaService_ReadVbmrPercent : FunctionCaller  FunctionPrototype=[Percent,ERR] = Lin2ParaService_ReadVbmrPercent()  OutputArgumentSpecifications=uint8(1), P_Std_ReturnType
  Lin2ParaService_VbmrReqPercentRetCtrltoECU : FunctionCaller  FunctionPrototype=ERR = Lin2ParaService_VbmrReqPercentRetCtrltoECU()  OutputArgumentSpecifications=P_Std_ReturnType
  Lin2ParaService_VbmrReqPercentShortTermAdj : FunctionCaller  FunctionPrototype=ERR = Lin2ParaService_VbmrReqPercentShortTermAdj(Percent)  InputArgumentSpecifications=uint8(1)  OutputArgumentSpecifications=P_Std_ReturnType
  LoadParaService_FreezeIncarReqStatus : FunctionCaller  FunctionPrototype=ERR = LoadParaService_FreezeIncarReqStatus()  OutputArgumentSpecifications=P_Std_ReturnType
  LoadParaService_FreezeReTXVReqStatus : FunctionCaller  FunctionPrototype=ERR = LoadParaService_FreezeReTXVReqStatus()  OutputArgumentSpecifications=P_Std_ReturnType
  LoadParaService_FreezeVlclPwmPercent : FunctionCaller  FunctionPrototype=ERR = LoadParaService_FreezeVlclPwmPercent()  OutputArgumentSpecifications=P_Std_ReturnType
  LoadParaService_FreezeVlclRearPwmPercent : FunctionCaller  FunctionPrototype=ERR = LoadParaService_FreezeVlclRearPwmPercent()  OutputArgumentSpecifications=P_Std_ReturnType
  LoadParaService_IncarReqRetCtrltoECU : FunctionCaller  FunctionPrototype=ERR = LoadParaService_IncarReqRetCtrltoECU()  OutputArgumentSpecifications=P_Std_ReturnType
  LoadParaService_IncarReqShortTermAdj : FunctionCaller  FunctionPrototype=ERR = LoadParaService_IncarReqShortTermAdj(Status)  InputArgumentSpecifications=uint8(1)  OutputArgumentSpecifications=P_Std_ReturnType
  LoadParaService_ReTXVReqRetCtrltoECU : FunctionCaller  FunctionPrototype=ERR = LoadParaService_ReTXVReqRetCtrltoECU()  OutputArgumentSpecifications=P_Std_ReturnType
  LoadParaService_ReTXVReqShortTermAdj : FunctionCaller  FunctionPrototype=ERR = LoadParaService_ReTXVReqShortTermAdj(Status)  InputArgumentSpecifications=uint8(1)  OutputArgumentSpecifications=P_Std_ReturnType
  LoadParaService_ReadIncarMotorStatus : FunctionCaller  FunctionPrototype=[Status,ERR] = LoadParaService_ReadIncarMotorStatus()  OutputArgumentSpecifications=uint8(1), P_Std_ReturnType
  LoadParaService_ReadReTXVStatus : FunctionCaller  FunctionPrototype=[Status,ERR] = LoadParaService_ReadReTXVStatus()  OutputArgumentSpecifications=uint8(1), P_Std_ReturnType
  LoadParaService_SetBlwFbTriggerFlg : FunctionCaller  FunctionPrototype=ERR = LoadParaService_SetBlwFbTriggerFlg()  OutputArgumentSpecifications=P_Std_ReturnType
  LoadParaService_VlclPwmRetCtrltoECU : FunctionCaller  FunctionPrototype=ERR = LoadParaService_VlclPwmRetCtrltoECU()  OutputArgumentSpecifications=P_Std_ReturnType
  LoadParaService_VlclPwmShortTermAdj : FunctionCaller  FunctionPrototype=ERR = LoadParaService_VlclPwmShortTermAdj(Duty)  InputArgumentSpecifications=uint8(1)  OutputArgumentSpecifications=P_Std_ReturnType
  LoadParaService_VlclReadBlwDuty : FunctionCaller  FunctionPrototype=[Duty,ERR] = LoadParaService_VlclReadBlwDuty()  OutputArgumentSpecifications=uint8(1), P_Std_ReturnType
  LoadParaService_VlclReadRearBlwDuty : FunctionCaller  FunctionPrototype=[Duty,ERR] = LoadParaService_VlclReadRearBlwDuty()  OutputArgumentSpecifications=uint8(1), P_Std_ReturnType
  LoadParaService_VlclRearPwmRetCtrltoECU : FunctionCaller  FunctionPrototype=ERR = LoadParaService_VlclRearPwmRetCtrltoECU()  OutputArgumentSpecifications=P_Std_ReturnType
  LoadParaService_VlclRearPwmShortTermAdj : FunctionCaller  FunctionPrototype=ERR = LoadParaService_VlclRearPwmShortTermAdj(Duty)  InputArgumentSpecifications=uint8(1)  OutputArgumentSpecifications=P_Std_ReturnType
  PwrParaService_GetMotorCailCnt : FunctionCaller  FunctionPrototype=[Cnt,ERR] = PwrParaService_GetMotorCailCnt(Channel)  InputArgumentSpecifications=PowerChlType(0)  OutputArgumentSpecifications=uint8(1), P_Std_ReturnType
  PwrParaService_GetMotorCailNum : FunctionCaller  FunctionPrototype=[Num,ERR] = PwrParaService_GetMotorCailNum(Channel)  InputArgumentSpecifications=PowerChlType(0)  OutputArgumentSpecifications=uint8(1), P_Std_ReturnType
  PwrParaService_SetMotorCailCnt : FunctionCaller  FunctionPrototype=ERR = PwrParaService_SetMotorCailCnt(Channel,Cnt)  InputArgumentSpecifications=PowerChlType(0), uint8(1)  OutputArgumentSpecifications=P_Std_ReturnType
  PwrParaService_SetMotorCailNum : FunctionCaller  FunctionPrototype=ERR = PwrParaService_SetMotorCailNum(Channel,Num)  InputArgumentSpecifications=PowerChlType(0), uint8(1)  OutputArgumentSpecifications=P_Std_ReturnType
  SensorParaService_GetValidValue : FunctionCaller  FunctionPrototype=[ValidValue,ERR] = SensorParaService_GetValidValue(ucChannel)  InputArgumentSpecifications=SrsAdChlType(0)  OutputArgumentSpecifications=uint16(1), P_Std_ReturnType
  SysServParaService_ReadCarConfigParaFaultCnt : FunctionCaller  FunctionPrototype=[Data,ERR] = SysServParaService_ReadCarConfigParaFaultCnt(NuM,type)  InputArgumentSpecifications=uint8(1), CCPFaultType(0)  OutputArgumentSpecifications=uint8([1;1]), P_Std_ReturnType
  SysServParaService_ReadData_DIDD005 : FunctionCaller  FunctionPrototype=[Data,ERR] = SysServParaService_ReadData_DIDD005()  OutputArgumentSpecifications=uint8([1;1;1;1;1;1;1;1;1;1;1;1;1;1;1;1;1;1;1;1;1;1;1;1;1;1;1;1;1;1;1;1;1;1;1;1;1;1;1;1;1;1;1;1;1;1;1;1;1;1;1;1;1;1;1;1;1;1;1;1;1;1;1;1;1;1;1;1;1;1;1;1;1;1;1;1;1;1;1;1;1;1;1;1;1;1;1;1;1;1]), P_Std_ReturnType
  SysServParaService_ReadData_DIDD09A : FunctionCaller  FunctionPrototype=[Data,ERR] = SysServParaService_ReadData_DIDD09A()  OutputArgumentSpecifications=uint8([1]), P_Std_ReturnType
  SysServParaService_ReadData_DIDF190 : FunctionCaller  FunctionPrototype=[Data,ERR] = SysServParaService_ReadData_DIDF190()  OutputArgumentSpecifications=uint8([1;1;1;1;1;1;1;1;1;1;1;1;1;1;1;1]), P_Std_ReturnType
  SysServParaService_ReadData_DIDF1AB : FunctionCaller  FunctionPrototype=[Data,ERR] = SysServParaService_ReadData_DIDF1AB()  OutputArgumentSpecifications=uint8([1;1;1;1;1;1;1;1]), P_Std_ReturnType
  SysServParaService_WriteData_DIDD09A : FunctionCaller  FunctionPrototype=ERR = SysServParaService_WriteData_DIDD09A(Data)  InputArgumentSpecifications=uint8([1])  OutputArgumentSpecifications=P_Std_ReturnType
  SysServParaService_WriteData_DIDF1AB : FunctionCaller  FunctionPrototype=ERR = SysServParaService_WriteData_DIDF1AB(Part,Data)  InputArgumentSpecifications=P_SignalPartType, uint8([1;1;1;1;1;1;1;1])  OutputArgumentSpecifications=P_Std_ReturnType
  Terminator_{1..497} (497 alike) : Terminator
  VgaCaliServive_SetAllCalibrate : FunctionCaller  FunctionPrototype=ERR = VgaCaliServive_SetAllCalibrate()  OutputArgumentSpecifications=P_Std_ReturnType
  VgaCaliServive_SetAllParaInit : FunctionCaller  FunctionPrototype=ERR = VgaCaliServive_SetAllParaInit()  OutputArgumentSpecifications=P_Std_ReturnType
  VgaCaliServive_SetMotorCalibrate : FunctionCaller  FunctionPrototype=ERR = VgaCaliServive_SetMotorCalibrate(Channel)  InputArgumentSpecifications=VGAChlType(0)  OutputArgumentSpecifications=P_Std_ReturnType
  VgaCaliServive_SetMotorParaInit : FunctionCaller  FunctionPrototype=ERR = VgaCaliServive_SetMotorParaInit(Channel)  InputArgumentSpecifications=VGAChlType(0)  OutputArgumentSpecifications=P_Std_ReturnType
  VgaDtcService_GetElecErr : FunctionCaller  FunctionPrototype=[Status,ERR] = VgaDtcService_GetElecErr(Channel)  InputArgumentSpecifications=VGAChlType(0)  OutputArgumentSpecifications=uint8(1), P_Std_ReturnType
  VgaDtcService_GetRecErr : FunctionCaller  FunctionPrototype=[Status,ERR] = VgaDtcService_GetRecErr(Channel)  InputArgumentSpecifications=VGAChlType(0)  OutputArgumentSpecifications=uint8(1), P_Std_ReturnType
  VgaDtcService_GetStallErr : FunctionCaller  FunctionPrototype=[Status,ERR] = VgaDtcService_GetStallErr(Channel)  InputArgumentSpecifications=VGAChlType(0)  OutputArgumentSpecifications=uint8(1), P_Std_ReturnType
  VgaDtcService_GetTempErr : FunctionCaller  FunctionPrototype=[Status,ERR] = VgaDtcService_GetTempErr(Channel)  InputArgumentSpecifications=VGAChlType(0)  OutputArgumentSpecifications=uint8(1), P_Std_ReturnType
  VgaDtcService_GetVoltErr : FunctionCaller  FunctionPrototype=[Status,ERR] = VgaDtcService_GetVoltErr(Channel)  InputArgumentSpecifications=VGAChlType(0)  OutputArgumentSpecifications=uint8(1), P_Std_ReturnType